simulink model "TosAeb"  (R2021a)
settings: Solver=FixedStepDiscrete  StartTime=0.0  StopTime=inf  FixedStep=0.01  RelTol=1e-3
undefined symbols (not defined in the file or in its folder): ACCModSts, AccSts1, AEB_ObjectMovingOrientation, AEB_ObjectMovingState, CN_BTCActive, CN_CurrentGearR, CN_CurrentGearR_DHT, CN_PTCActive, CN_VDCActive, CN_VSC_EspOff, CN_VSC_FCW_AEB_Error, CN_VSC_FCW_AEB_NoDispay, CN_VSC_FCW_AEB_PerfmDgdtn, CN_VSC_FCW_AEB_TempUnAvlbl, CN_VSC_VehStandstill, CNaccelAvoidMax, CNaccelAvoidMin, CNbrakePedalPress, CNcomfortStrLeftAccelMax, CNcomfortStrLeftAccelMin, CNcomfortStrRightAccelMax, CNcomfortStrRightAccelMin, CNDrvDoorOpen, CNDrvModeEco, CNDrvModeNorm, CNDrvModeOther, CNDrvModeSport, CNDrvSBRreminder, CNNull, CNobjInvalidID, CNPassDoorOpen, CNPassSBRreminder, CNPEBSAccelNegInf, CNPEBSAccelNull, CNPEBSTimeInf, CNPEBSTimeNull, CNSTOPMAX, CNSTOPNULL, CNsubjWidth, CNSwtOFF, CNSwtON, CNTTCMAX, CNTTCNULL, CNVehConfigAEBCycNone, CNVehConfigAEBNone, CNVehConfigAEBPedNone, CNVehConfigFCWNone, CNVehConfigJANone, CollisionSeverity, FALSE, FCWSnvty, JACycObjCalcInfoDefaultParams, JAPedObjCalcInfoDefaultParams, JAVehObjCalcInfoDefaultParams, latCycObjCalcInfoDefaultParams, latPedObjCalcInfoDefaultParams, longObjCalcInfoDefaultParams, ModSts, movingStatus, ObjectType ... (+1112)
libraries: simulink x3031, AEBSFunctionLib x50, Ta_Library x33, AEBSStateMachineLib x23, Mylibrary x12, simulink_extras x7, ObjSelect_lib x3, dspstat3 x2
(part 97 of 102)

== stateflow chart TosAeb/FcwAebJa_Step_sys/ALG_AEBSystem/ALG_AEBController/AEB_FuncCondCalcApp/PedFunctionCondCalc/pedPrefillSuppAborCond/pedPrefillSuppCondCalc/pedPrefillSituationRelActiveCondCalc/objLatVyCond4PrefillCalc/ObjectIsLaterallyMovingForPrefillCondCalc ==
  (default) -> (junction 4)
  (junction 4) -> (junction 5) [order 1] : [abs(vyCorr) < P_pedPrefillVyMaxThres]
  (junction 4) -> (junction 23) [order 2] : {
      | 	objLatVyCond4Prefill = false;
      | }
  (junction 5) -> (junction 7)
  (junction 7) -> (junction 9) [order 1] : [abs(dy)< P_pedPrefillDyInnerAreaThres]
  (junction 9) -> (junction 32)
  (junction 7) -> (junction 13) [order 2]
  (junction 32) -> (junction 34) [order 1] : [ObjectTypeCameraConfirmed == true]
  (junction 32) -> (junction 11) [order 2] : {
      | 	objLatVyCond4Prefill = ...
      | (abs(vyCorr) > P_pedPrefillVyInnerAreaThres4Radar);
      | }
  (junction 34) -> (junction 36) : {
      | 	objLatVyCond4Prefill = ...
      | (abs(vyCorr) > P_pedPrefillVyInnerAreaThres4Fusion);
      | }
  (junction 13) -> (junction 15) [order 1] : [abs(dy)<P_pedPrefillDyMiddleAreaThres]
  (junction 13) -> (junction 48) [order 2]
  (junction 15) -> (junction 39)
  (junction 39) -> (junction 41) [order 1] : [ObjectTypeCameraConfirmed == true]
  (junction 39) -> (junction 43) [order 2] : {
      | 	objLatVyCond4Prefill = ...
      | (abs(vyCorr) > P_pedPrefillVyMiddleAreaThres4Radar);
      | }
  (junction 41) -> (junction 17) : {
      | 	objLatVyCond4Prefill = ...
      | (abs(vyCorr) > P_pedPrefillVyMiddleAreaThres4Fusion);
      | }
  (junction 48) -> (junction 50) [order 1] : [ObjectTypeCameraConfirmed == true]
  (junction 48) -> (junction 19) [order 2] : {
      | 	objLatVyCond4Prefill = ...
      | (abs(vyCorr) > P_pedPrefillVyOuterAreaThres4Radar);
      | }
  (junction 50) -> (junction 46) : {
      | 	objLatVyCond4Prefill = ...
      | (abs(vyCorr) > P_pedPrefillVyOuterAreaThres4Fusion);
      | }
  (junction 36) -> (junction 11)
  (junction 11) -> (junction 17)
  (junction 19) -> (junction 23)
  (junction 46) -> (junction 19)
  (junction 43) -> (junction 46)
  (junction 17) -> (junction 43)
  (junction 23) -> (junction 26)
  data: ObjectTypeCameraConfirmed input; dy input; vyCorr input; P_pedPrefillVyMaxThres input; objLatVyCond4Prefill output; P_pedPrefillDyInnerAreaThres input; P_pedPrefillDyMiddleAreaThres input; P_pedPrefillVyInnerAreaThres4Fusion input; P_pedPrefillVyInnerAreaThres4Radar input; P_pedPrefillVyMiddleAreaThres4Fusion input; P_pedPrefillVyMiddleAreaThres4Radar input; P_pedPrefillVyOuterAreaThres4Fusion input; P_pedPrefillVyOuterAreaThres4Radar input

== stateflow chart TosAeb/FcwAebJa_Step_sys/ALG_AEBSystem/ALG_AEBController/AEB_FuncImpManagementApp/FuncBlockTimeCalc/tBlockTimeBrkCalc/BlockTimeCalc/BlockTimeCalc ==
  state A
    en,du:
    blockTime = 0;
  state B
    en:
    blockTime = PblockTime;
    du:
    blockTime -= Ts;
  (default) -> (junction 7)
  (junction 7) -> A [order 2]
  (junction 7) -> B [order 1] : [blockTimeTriggerCond == true]
  B -> A : [blockTime <= 0]
  A -> B : [blockTimeTriggerCond == true]
  data: blockTimeTriggerCond input; Ts input single; PblockTime input single; blockTime output single

== stateflow chart TosAeb/FcwAebJa_Step_sys/ALG_AEBSystem/ALG_AEBController/AEB_FuncImpManagementApp/FuncBlockTimeCalc/tBlockTimeBrkReadyCalc/BlockTimeCalc/BlockTimeCalc ==
  state A
    en,du:
    blockTime = 0;
  state B
    en:
    blockTime = PblockTime;
    du:
    blockTime -= Ts;
  (default) -> (junction 7)
  (junction 7) -> A [order 2]
  (junction 7) -> B [order 1] : [blockTimeTriggerCond == true]
  B -> A : [blockTime <= 0]
  A -> B : [blockTimeTriggerCond == true]
  data: blockTimeTriggerCond input; Ts input single; PblockTime input single; blockTime output single

== stateflow chart TosAeb/FcwAebJa_Step_sys/ALG_AEBSystem/ALG_AEBController/AEB_FuncImpManagementApp/FuncBlockTimeCalc/tBlockTimeWarningCalc/BlockTimeCalc/BlockTimeCalc ==
  state A
    en,du:
    blockTime = 0;
  state B
    en:
    blockTime = PblockTime;
    du:
    blockTime -= Ts;
  (default) -> (junction 7)
  (junction 7) -> A [order 2]
  (junction 7) -> B [order 1] : [blockTimeTriggerCond == true]
  B -> A : [blockTime <= 0]
  A -> B : [blockTimeTriggerCond == true]
  data: blockTimeTriggerCond input; Ts input single; PblockTime input single; blockTime output single

== stateflow chart TosAeb/FcwAebJa_Step_sys/ALG_AEBSystem/ALG_AEBController/AEB_FuncImpManagementApp/FuncManeuArbCalc/BrkReadyManeuArbCalc/BrkReadyTriggerJudge/ABALvlCalc/If Action Subsystem/ABALvlCalcFlow ==
  state ABATriggerState
    state ABALvl3
      en,du:
      ABALvl = uint8(3);
    state ABALvl2
      en,du:
      ABALvl = uint8(2);
    state ABALvl1
      en,du:
      ABALvl = uint8(1);
    (default) -> (junction 14)
    (junction 14) -> ABALvl3 [order 1] : [AEB_CollisionSeverity == CollisionSeverity.High]
    (junction 14) -> (junction 16) [order 2]
    (junction 58) -> ABALvl3
    (junction 56) -> (junction 58)
    ABALvl2 -> ABALvl3 : [AEB_CollisionSeverity == CollisionSeverity.High]
    (junction 16) -> ABALvl2 [order 1] : [AEB_CollisionSeverity == CollisionSeverity.Medium]
    (junction 16) -> (junction 19) [order 2]
    ABALvl1 -> ABALvl2 [order 2] : [AEB_CollisionSeverity == CollisionSeverity.Medium]
    (junction 19) -> ABALvl1
    ABALvl1 -> (junction 56) [order 1] : [AEB_CollisionSeverity == CollisionSeverity.High]
  state ABANoTriggerState
    en,du:
    ABALvl = 0;
  (default) -> ABANoTriggerState
  ABANoTriggerState -> ABATriggerState : [ABATrigger == true && ...
      | AEB_CollisionSeverity ~= CollisionSeverity.None]
  ABATriggerState -> ABANoTriggerState : [ABATrigger == false]
  data: ABATrigger input; AEB_CollisionSeverity input Enum: CollisionSeverity; ABALvl output uint8

== stateflow chart TosAeb/FcwAebJa_Step_sys/ALG_AEBSystem/ALG_AEBController/AEB_FuncImpManagementApp/FuncManeuArbCalc/WarningManeuArbCalc/WarningTriggerCalc/acuteWarningLvlCalcFlow ==
  state acuteWarningTriggerState
    state Lvl4
      en,du:
      actuteWarningLvl = uint8(4);
    state Lvl3
      en,du:
      actuteWarningLvl = uint8(3);
    state Lvl2
      en,du:
      actuteWarningLvl = uint8(2);
    state Lvl1
      en,du:
      actuteWarningLvl = uint8(1);
    (default) -> (junction 54)
    (junction 54) -> Lvl4 [order 1] : [subjVel > P_FIM_AcutewarningLvl4VehSpdMinThres ]
    (junction 54) -> (junction 56) [order 2]
    (junction 56) -> Lvl3 [order 1] : [subjVel > P_FIM_AcutewarningLvl3VehSpdMinThres]
    (junction 56) -> (junction 59) [order 2]
    (junction 59) -> Lvl2 [order 1] : [subjVel > P_FIM_AcutewarningLvl2VehSpdMinThres]
    (junction 59) -> (junction 62) [order 2]
    (junction 62) -> Lvl1
  state acuteWarningNoTrigger
    en,du:
    actuteWarningLvl = uint8(0);
  (default) -> acuteWarningNoTrigger
  acuteWarningNoTrigger -> acuteWarningTriggerState : [acuteWarningTrigger == true &&...
      | subjVel>=P_FIM_AcutewarningLvl1VehSpdMinThres&&...
      | subjVel<=P_FIM_AcutewarningLvl4VehSpdMaxThres]
  acuteWarningTriggerState -> acuteWarningNoTrigger : [acuteWarningTrigger == false]
  data: acuteWarningTrigger input boolean; subjVel input; actuteWarningLvl output uint8; P_FIM_AcutewarningLvl1VehSpdMinThres input; P_FIM_AcutewarningLvl2VehSpdMinThres input; P_FIM_AcutewarningLvl3VehSpdMinThres input; P_FIM_AcutewarningLvl4VehSpdMinThres input; P_FIM_AcutewarningLvl4VehSpdMaxThres input

== matlab function TosAeb/AEB_Objsel/TA_Processor/AEB_ObjSelect/TargetInfoCheck/Data_precess/TA_EffectCalculate/Obj_FilterFlgCalculate/JA_ObjFilter/CollisionProbCalc/Subsystem/Subsystem/SegmentComponents/LatPosition ==
  data: P0 input; y output single; P1 input; P2 input; P3 input; tau input; dy output single
function [y, dy] = fun(P0,P1,P2,P3,tau)
%#codegen

%TODO Description is missing 

%For performance reasons the original expression, below, has been rewritten  

%Original expressions 
% dx为x的对自变量tau的导数
% 式中x为距离，dx为速度
% 式中tau为x取值对应的概率值，p0，p1,p2,p3前系数值的和为"1"
%x=(1-tau)^3*P0 + 3*(1-tau)^2*tau*P1 + 3*(1-tau)*tau.^2*P2 + tau^3*P3;
%dx = -3*(1-tau)^2*P0 + 3*(3*tau^2 - 4*tau + 1)*P1 + 3*(2*tau - 3*tau^2)*P2 + 3*tau^2*P3; 

tauPov2 = tau*tau; 
tauPov3 = tau*tauPov2; 
x = (3*(P1 - P2) - P0 + P3)*tauPov3 + 3*(P0 - 2*P1 + P2)*tauPov2 + 3*(P1 - P0)*tau + P0; 
dx = (9*P1 - 3*P0 - 9*P2 + 3*P3)*tauPov2 + (6*P0 - 12*P1 + 6*P2)*tau - 3*P0 + 3*P1;
y = x;
dy = dx;

== matlab function TosAeb/AEB_Objsel/TA_Processor/AEB_ObjSelect/TargetInfoCheck/Data_precess/TA_EffectCalculate/Obj_FilterFlgCalculate/JA_ObjFilter/CollisionProbCalc/Subsystem/Subsystem/SegmentComponents/LongPosition ==
  data: P0 input; x output single; P1 input; P2 input; P3 input; tau input; dx output single
function [x,dx] = fcn(P0,P1,P2,P3,tau)
%#codegen

%TODO Description is missing 

%For performance reasons the original expression, below, has been rewritten  

%Original expressions 
% dx为x的对自变量tau的导数
% 式中x为距离，dx为速度
% 式中tau为x取值对应的概率值，p0，p1,p2,p3前系数值的和为"1"
%x=(1-tau)^3*P0 + 3*(1-tau)^2*tau*P1 + 3*(1-tau)*tau.^2*P2 + tau^3*P3;
%dx = -3*(1-tau)^2*P0 + 3*(3*tau^2 - 4*tau + 1)*P1 + 3*(2*tau - 3*tau^2)*P2 + 3*tau^2*P3; 

tauPov2 = tau*tau; 
tauPov3 = tau*tauPov2; 
x = (3*(P1 - P2) - P0 + P3)*tauPov3 + 3*(P0 - 2*P1 + P2)*tauPov2 + 3*(P1 - P0)*tau + P0; 
dx = (9*P1 - 3*P0 - 9*P2 + 3*P3)*tauPov2 + (6*P0 - 12*P1 + 6*P2)*tau - 3*P0 + 3*P1;

== stateflow chart TosAeb/AEB_Objsel/TA_Processor/AEB_ObjSelect/TargetInfoCheck/Data_precess/TA_EffectCalculate/Obj_FilterFlgCalculate/JA_ObjFilter/CollisionProbCalcFlow ==
  (default) -> (junction 11)
  (junction 11) -> (junction 2) : {tPredictTime = 0;}
  (junction 2) -> (junction 6) [order 1] : [tPredictTime <= P_JaPedCA_PreCollisionPredTimeMax]
  (junction 6) -> (junction 8) : {
      | 	tPredictTimeOut = tPredictTime;
      | 	CollisionProbFunc;
      | }
  (junction 8) -> (junction 2) [order 2] : {
      | 	tPredictTime += P_JaPedCA_PreCollisionStepTime;
      | }
  (junction 2) -> (junction 13) [order 2] : {
      |   TTC = single(256);
      | }
  (junction 8) -> (junction 22) [order 1] : [preCollisionProb == true]
  (junction 22) -> (junction 13) : {
      |   TTC = tPredictTimeOut;
      | }
  (junction 13) -> (junction 25)
  data: TTC output single; P_JaPedCA_PreCollisionStepTime input single; P_JaPedCA_PreCollisionPredTimeMax input single; tPredictTimeOut output single; tPredictTime local single; preCollisionProb input
  events: CollisionProbFunc output FUNCTION_CALL_EVENT

== stateflow chart TosAeb/FcwAebJa_Step_sys/ALG_AEBSystem/ALG_AEBController/AEB_FuncImpManagementApp/FuncManeuArbCalc/brkFuncManeuArbCalc/axTgtReqValueManeuCalc/AEBTriggerManeuCalc ==
  state AEBQuitState
    en:
    timeCounter = 0;
    AEBTriggerManeu = true;
    %axTgtReqValueIntial = axTgtReqValue;
    du:
    AEBTriggerManeu = true;
    timeCounter += Ts;
    axTgtReqValueManeu = axTgtReqValueOptCalc(Ts,axJerkQuit,axTgtReqValueManeuK1);
  state AEBNormalState
    en,du:
    timeCounter = 0;
    AEBTriggerManeu= AEBTrigger;
    axTgtReqValueManeu = axTgtReqValue;
    %exit:
    %axTgtReqValueIntial = axTgtReqValueK1;
  state axTgtReqValueManeu = axTgtReqValueOptCalc(Ts,axJerkQuit,axTgtReqValueManeuK1) [function]
  (default) -> (junction 7)
  (junction 7) -> AEBNormalState [order 2]
  (junction 7) -> AEBQuitState [order 1] : [AEBQuitFlg == true]
  AEBQuitState -> AEBNormalState : [timeCounter >= PmaxQuitTime || ...
      | abs(axTgtReqValueManeu) < 1e-6]
  AEBNormalState -> AEBQuitState : [AEBQuitFlg == true]
  data: Ts function_input single; axJerkQuit function_input single; axTgtReqValueManeuK1 function_input single; axTgtReqValueManeu function_output; AEBQuitFlg input; AEBTrigger input; axTgtReqValue input single; Ts input single; timeCounter local single; PmaxQuitTime input single; AEBTriggerManeu output boolean; axTgtReqValueManeu output single; axJerkQuit input single; axTgtReqValueManeuK1 input

== stateflow chart TosAeb/FcwAebJa_Step_sys/ALG_AEBSystem/ALG_AEBController/AEB_FuncImpManagementApp/FuncManeuArbCalc/brkFuncManeuArbCalc/brkManeuAborCalc/ESPRelAborCondCalc/ESPRelAborCondFlow ==
  (default) -> (junction 6)
  (junction 6) -> (junction 7) [order 1] : [AEBIBK1 == true]
  (junction 6) -> (junction 18) [order 2]
  (junction 7) -> (junction 13) : {
      | 	ESPRelAborCond = ((AEBIB == false) &&(AEBBA == false));
      | }
  (junction 18) -> (junction 20) [order 1] : [AEBBAK1 == true]
  (junction 18) -> (junction 24) [order 2] : {
      | 	ESPRelAborCond = false;
      | }
  (junction 20) -> (junction 22) : {
      | 	ESPRelAborCond = (AEBBA == false);
      | }
  (junction 22) -> (junction 24)
  (junction 13) -> (junction 22)
  (junction 24) -> (junction 28)
  data: AEBBA input; AEBBAK1 input; AEBIB input; AEBIBK1 input; ESPRelAborCond output

== stateflow chart TosAeb/FcwAebJa_Step_sys/ALG_AEBSystem/ALG_AEBController/AEB_FuncImpManagementApp/FuncManeuArbCalc/brkFuncManeuArbCalc/brkManeuAborCalc/VelReduceMaxCond/vxTriggerCalc ==
  state VelReduceMaxCondCalc [function]
    (default) -> (junction 38)
    (junction 38) -> (junction 40) [order 1] : [EBAFuncSts == ModSts.ActiveState]
    (junction 38) -> (junction 63) [order 2]
    (junction 40) -> (junction 42)
    (junction 63) -> (junction 65) [order 1] : [vxTrigger < P_FIM_AEBVelDecMaxThres]
    (junction 42) -> (junction 45) [order 1] : [vxTrigger < P_FIM_EBAVelDecMaxThres]
    (junction 63) -> (junction 69) [order 2] : {
        | subjVelReduced = ...
        | vxTrigger - P_FIM_AEBVelDecMaxThres;
        | }
    (junction 65) -> (junction 67) : {
        |   subjVelReduced  = 0;
        | }
    (junction 42) -> (junction 49) [order 2] : {
        | subjVelReduced = ...
        | vxTrigger - P_FIM_EBAVelDecMaxThres;
        | }
    (junction 45) -> (junction 47) : {
        |   subjVelReduced  = 0;
        | }
    (junction 67) -> (junction 69)
    (junction 69) -> (junction 88)
    (junction 47) -> (junction 49)
    (junction 49) -> (junction 86)
    (junction 86) -> (junction 88)
    (junction 88) -> (junction 52)
    (junction 52) -> (junction 54) [order 1] : [subjVel < subjVelReduced]
    (junction 52) -> (junction 58) [order 2] : {
        |   VelReduceMaxCond = false;
        | }
    (junction 54) -> (junction 56) : {
        |   VelReduceMaxCond = true;
        | }
    (junction 56) -> (junction 58)
    (junction 58) -> (junction 61)
  state PassiveState
    en,du:
    %vxTrigger = 0;
    VelReduceMaxCond = false;
  state TriggerState
    en:
    vxTrigger = max(subjVel,0);
    du:
    VelReduceMaxCondCalc();
  (default) -> PassiveState
  PassiveState -> TriggerState : [AEBTrigger == true]
  TriggerState -> PassiveState : [AEBTrigger == false]
  data: AEBTrigger input boolean; subjVel input single; EBAFuncSts input Enum: ModSts; vxTrigger local single; VelReduceMaxCond output boolean; P_FIM_EBAVelDecMaxThres input single; P_FIM_AEBVelDecMaxThres input single; subjVelReduced local single

== stateflow chart TosAeb/FcwAebJa_Step_sys/ALG_AEBSystem/ALG_AEBController/AEB_LongTgtInfoCalc/LongObjCalcInfoCalc/AEBS_LongCollisionAssesApp/DriverReactionTimeCalc/ACCStateMonitor/ACCStateMonitor ==
  state NormalState
    en,du:
    NormalStateFunc();
  state NormalStateFunc [function]
    (default) -> (junction 86)
    (junction 86) -> (junction 84) [order 1] : [ACCstateK1 == 0 &&...
        | ACCstate == 1]
    (junction 86) -> (junction 80) [order 2] : {
        | 	ACCMonitor = false;
        | }
    (junction 84) -> (junction 79) : {
        | ACCMonitor = true;
        | }
    (junction 79) -> (junction 80)
    (junction 80) -> (junction 89)
  state CounterState
    en:
    timesincelastACC = 0;
    du:
    timesincelastACC +=  Ts;
    CounterStateFunc();
  state CounterStateFunc [function]
    (default) -> (junction 70)
    (junction 70) -> (junction 62) [order 1] : [timesincelastACC > CNreactionTimetimesincelastACCMAX]
    (junction 70) -> (junction 69) [order 2] : %do nothing
    (junction 62) -> (junction 61) : {
        | timesincelastACC = CNreactionTimetimesincelastACCMAX;
        | }
    (junction 61) -> (junction 69)
    (junction 69) -> (junction 68)
    (junction 68) -> (junction 60) [order 1] : [timesincelastACC < PreactionTimeProlongationTimeACC]
    (junction 68) -> (junction 66) [order 2] : {
        | ACCMonitor = false;
        | }
    (junction 60) -> (junction 67) : {
        | ACCMonitor = true;
        | }
    (junction 67) -> (junction 66)
    (junction 66) -> (junction 56)
  (default) -> NormalState
  NormalState -> CounterState : [ACCstateK1 == true &&...
      | ACCstate == false]
  CounterState -> NormalState : [timesincelastACC >= PreactionTimeProlongationTimeACC]
  data: ACCstate input; ACCstateK1 input; PreactionTimeProlongationTimeACC input; timesincelastACC output double; ACCMonitor output boolean; Ts input; CNreactionTimetimesincelastACCMAX parameter double

== stateflow chart TosAeb/FcwAebJa_Step_sys/ALG_AEBSystem/ALG_AEBController/AEB_LongTgtInfoCalc/LongObjCalcInfoCalc/AEBS_LongCollisionAssesApp/DriverReactionTimeCalc/ReationTimeCalc1/ReactionTimeLevel1Calc ==
  note: Diver Reaction Time Calculation
  note: Note:HMISetting Code
      | CNHMIEarly: 0
      | CNHMIMiddle: 1
      | CNHMILate: 2
  (default) -> (junction 7)
  (junction 7) -> (junction 8) [order 1] : [FcwHMISetting == FCWSnvty.HMISettingEarly]
  (junction 7) -> (junction 19) [order 2]
  (junction 8) -> (junction 10)
  (junction 19) -> (junction 21) [order 1] : [FcwHMISetting == FCWSnvty.HMISettingMiddle]
  (junction 19) -> (junction 39) [order 2] : %HMISetting == CNHMILate
  (junction 21) -> (junction 23)
  (junction 39) -> (junction 41) [order 1] : [driverDetectAsActiveFlag == true]
  (junction 23) -> (junction 32) [order 1] : [driverDetectAsActiveFlag == true]
  (junction 10) -> (junction 12) [order 1] : [driverDetectAsActiveFlag == true]
  (junction 39) -> (junction 45) [order 2] : {
      | 	tempDiverReactionTimeLvl1 = ...
      | 	PreactionTimeLvl1HMILate;
      | }
  (junction 41) -> (junction 43) : {
      | 	tempDiverReactionTimeLvl1 = ...
      | 	PreactionTimeAttentiveDriverLvl1HMILate;
      | }
  (junction 23) -> (junction 30) [order 2] : {
      | 	tempDiverReactionTimeLvl1 = ...
      | 	PreactionTimeLvl1HMIMiddle;
      | }
  (junction 32) -> (junction 34) : {
      | 	tempDiverReactionTimeLvl1 = ...
      | 	PreactionTimeAttentiveDriverLvl1HMIMiddle;
      | }
  (junction 10) -> (junction 16) [order 2] : {
      | 	tempDiverReactionTimeLvl1 = ...
      | 	PreactionTimeLvl1HMIEarly;
      | }
  (junction 12) -> (junction 14) : {
      | 	tempDiverReactionTimeLvl1 = ...
      | 	PreactionTimeAttentiveDriverLvl1HMIEarly;
      | }
  (junction 14) -> (junction 16)
  (junction 34) -> (junction 30)
  (junction 16) -> (junction 56)
  (junction 30) -> (junction 52)
  (junction 43) -> (junction 45)
  (junction 45) -> (junction 50)
  (junction 56) -> (junction 52)
  (junction 52) -> (junction 50)
  (junction 50) -> (junction 48) : {
      | 	diverReactionTimeLvl1 = tempDiverReactionTimeLvl1;
      |  }
  data: FcwHMISetting input; driverDetectAsActiveFlag input; PreactionTimeLvl1HMIEarly input; PreactionTimeLvl1HMIMiddle input; PreactionTimeLvl1HMILate input; PreactionTimeAttentiveDriverLvl1HMIEarly input; PreactionTimeAttentiveDriverLvl1HMIMiddle input; PreactionTimeAttentiveDriverLvl1HMILate input; diverReactionTimeLvl1 output; tempDiverReactionTimeLvl1 local double

== stateflow chart TosAeb/FcwAebJa_Step_sys/ALG_AEBSystem/ALG_AEBController/AEB_LongTgtInfoCalc/LongObjCalcInfoCalc/AEBS_LongCollisionAssesApp/LatCollisionAvoidCalc/AccelLatAvoidCalc4SO/accelLatAvoidLeftCalc/CalculateAccelSteeringLeft/CalculateAccelSteeringLeftFlow ==
  (default) -> (junction 2)
  (junction 2) -> (junction 3) [order 1] : [tempFlg]
  (junction 2) -> (junction 23) [order 2] : {
      | 	accelSteeringLeft  = CNcomfortStrLeftAccelMax;
      | }
  (junction 3) -> (junction 21) : {
      | 	tempData = PcomfortStrLatDistMinSO + dyRelTargetObjCalc;
      | }
  (junction 21) -> (junction 29) [order 1] : [tempData <= 0]
  (junction 21) -> (junction 33) [order 2] : {
      | 	accelSteeringLeftCalcFunc;
      | 	accelSteeringLeft = accelSteeringLeftIn;
      | }
  (junction 29) -> (junction 31) : {
      | 	accelSteeringLeft  = CNcomfortStrLeftAccelMin;
      | }
  (junction 33) -> (junction 23)
  (junction 31) -> (junction 33)
  (junction 23) -> (junction 46)
  data: tempFlg input; PcomfortStrLatDistMinSO input; dyRelTargetObjCalc input; accelSteeringLeftIn input; accelSteeringLeft output; tempData local; CNcomfortStrLeftAccelMax parameter; CNcomfortStrLeftAccelMin parameter
  events: accelSteeringLeftCalcFunc output FUNCTION_CALL_EVENT

== stateflow chart TosAeb/FcwAebJa_Step_sys/ALG_AEBSystem/ALG_AEBController/AEB_LongTgtInfoCalc/LongObjCalcInfoCalc/AEBS_LongCollisionAssesApp/LatCollisionAvoidCalc/AccelLatAvoidCalc4SO/accelLatAvoidRightCalc/CalculateAccelSteeringRight/CalculateAccelSteeringLeftFlow ==
  (default) -> (junction 2)
  (junction 2) -> (junction 3) [order 1] : [tempFlg]
  (junction 2) -> (junction 23) [order 2] : {
      | 	accelSteeringRight  = CNcomfortStrRightAccelMin;
      | }
  (junction 3) -> (junction 21) : {
      | 	tempData = PcomfortStrLatDistMinSO - dyRelTargetObjCalc;
      | }
  (junction 21) -> (junction 29) [order 1] : [tempData <= 0]
  (junction 21) -> (junction 33) [order 2] : {
      | 	accelSteeringRightCalcFunc;
      | 	accelSteeringRight = accelSteeringRightIn;
      | }
  (junction 29) -> (junction 31) : {
      | 	accelSteeringRight  = CNcomfortStrRightAccelMax;
      | }
  (junction 33) -> (junction 23)
  (junction 31) -> (junction 33)
  (junction 23) -> (junction 46)
  data: tempFlg input; PcomfortStrLatDistMinSO input; dyRelTargetObjCalc input; tempData local; CNcomfortStrRightAccelMax parameter; CNcomfortStrRightAccelMin parameter; accelSteeringRightIn input; accelSteeringRight output
  events: accelSteeringRightCalcFunc output FUNCTION_CALL_EVENT

== stateflow chart TosAeb/FcwAebJa_Step_sys/ALG_AEBSystem/ALG_AEBController/AEB_LongTgtInfoCalc/LongObjCalcInfoCalc/AEBS_LongCollisionAssesApp/LongCollisionAvoidAssess/HighLvlBrkCalc/HighLvlBrkCalc4Brake/accelAvoidHighLvlBrkSituation1/accelAvoidLowLvlBrkCalc/AccelAvoidSituationJudge ==
  (default) -> (junction 16)
  (junction 16) -> (junction 17) [order 1] : [vx < 0]
  (junction 16) -> (junction 27) [order 2]
  (junction 17) -> (junction 74)
  (junction 74) -> (junction 76) [order 1] : [axAbsObj  < 0]
  (junction 27) -> (junction 29) [order 1] : [ axAbsObj  < 0]
  (junction 74) -> (junction 25) [order 2] : {
      | 	send(Situation2);
      | }
  (junction 76) -> (junction 19) : {
      | 	send(Situation1);
      | }
  (junction 27) -> (junction 33) [order 2] : {
      | 	send(Situation4);
      | }
  (junction 29) -> (junction 31) : {
      | 	send(Situation3);
      | }
  (junction 31) -> (junction 33)
  (junction 25) -> (junction 31)
  (junction 19) -> (junction 25)
  (junction 33) -> (junction 86)
  data: vx input; axAbsObj input
  events: Situation1 output FUNCTION_CALL_EVENT; Situation2 output FUNCTION_CALL_EVENT; Situation3 output FUNCTION_CALL_EVENT; Situation4 output FUNCTION_CALL_EVENT

== stateflow chart TosAeb/FcwAebJa_Step_sys/ALG_AEBSystem/ALG_AEBController/AEB_LongTgtInfoCalc/LongObjCalcInfoCalc/AEBS_LongCollisionAssesApp/LongCollisionAvoidAssess/HighLvlBrkCalc/HighLvlBrkCalc4Brake/accelAvoidHighLvlBrkSituation1/accelAvoidLowLvlBrkCalc2/AccelAvoidSituationJudge ==
  (default) -> (junction 16)
  (junction 16) -> (junction 17) [order 1] : [vxRelTargetObjCalc < 0 &&...
      |  axAbsTargetObjCalc  < 0]
  (junction 16) -> (junction 21) [order 2]
  (junction 17) -> (junction 19) : {send(Situation1);}
  (junction 21) -> (junction 23) [order 1] : [vxRelTargetObjCalc < 0 &&...
      |  axAbsTargetObjCalc  >= 0]
  (junction 21) -> (junction 27) [order 2]
  (junction 23) -> (junction 25) : {send(Situation2);}
  (junction 27) -> (junction 29) [order 1] : [vxRelTargetObjCalc > 0 &&...
      |  axAbsTargetObjCalc  < 0]
  (junction 27) -> (junction 33) [order 2] : {send(Situation4);}
  (junction 29) -> (junction 31) : {send(Situation3);}
  (junction 31) -> (junction 33)
  (junction 25) -> (junction 31)
  (junction 19) -> (junction 25)
  (junction 33) -> (junction 39)
  data: vxRelTargetObjCalc input; axAbsTargetObjCalc input
  events: Situation1 output FUNCTION_CALL_EVENT; Situation2 output FUNCTION_CALL_EVENT; Situation3 output FUNCTION_CALL_EVENT; Situation4 output FUNCTION_CALL_EVENT

== stateflow chart TosAeb/FcwAebJa_Step_sys/ALG_AEBSystem/ALG_AEBInput/LongObjInfoMap/ObjType_Subsystem/ObjectType ==
  (default) -> (junction 17)
  (junction 17) -> (junction 18) [order 1] : [ObjCar==1]
  (junction 17) -> (junction 19) [order 2]
  (junction 18) -> (junction 23) : {ObjType = 2;}
  (junction 19) -> (junction 20) [order 1] : [ObjTruck ==1]
  (junction 19) -> (junction 26) [order 2]
  (junction 20) -> (junction 22) : {
      | 	ObjType=3;
      | }
  (junction 26) -> (junction 27) [order 1] : [ObjPed==1]
  (junction 26) -> (junction 83) [order 2]
  (junction 27) -> (junction 29) : {
      | 	ObjType=6;
      | }
  (junction 83) -> (junction 85) [order 1] : [ObjMotor==1]
  (junction 83) -> (junction 91) [order 2]
  (junction 85) -> (junction 87) : {ObjType=4;}
  (junction 91) -> (junction 93) [order 1] : [ObjBicycle==1]
  (junction 91) -> (junction 100) [order 2]
  (junction 93) -> (junction 95) : {ObjType=5;}
  (junction 100) -> (junction 102) [order 1] : [ObjGeneral==1]
  (junction 100) -> (junction 21) [order 2] : {ObjType=1;}
  (junction 102) -> (junction 104) : {ObjType=7;}
  (junction 104) -> (junction 21)
  (junction 95) -> (junction 104)
  (junction 87) -> (junction 95)
  (junction 29) -> (junction 87)
  (junction 22) -> (junction 29)
  (junction 23) -> (junction 22)
  (junction 21) -> (junction 25)
  data: ObjCar input; ObjTruck input; ObjPed input; ObjType output uint8; ObjMotor input; ObjBicycle input; ObjGeneral input; Unknown input

== stateflow chart TosAeb/FcwAebJa_Step_sys/ALG_AEBSystem/ALG_AEBController/AEB_LongTgtInfoCalc/LongObjCalcInfoCalc/AEBS_LongCollisionAssesApp/LongCollisionAvoidAssess/HighLvlBrkCalc/HighLvlBrkCalc4Brake/accelAvoidHighLvlBrkSituation2/accelAvoidHighLvlBrkCalc/If Action Subsystem1/accelAvoidLowLvlBrkCalc/AccelAvoidSituationJudge ==
  (default) -> (junction 16)
  (junction 16) -> (junction 17) [order 1] : [vx < 0]
  (junction 16) -> (junction 27) [order 2]
  (junction 17) -> (junction 74)
  (junction 74) -> (junction 76) [order 1] : [axAbsObj  < 0]
  (junction 27) -> (junction 29) [order 1] : [ axAbsObj  < 0]
  (junction 74) -> (junction 25) [order 2] : {
      | 	send(Situation2);
      | }
  (junction 76) -> (junction 19) : {
      | 	send(Situation1);
      | }
  (junction 27) -> (junction 33) [order 2] : {
      | 	send(Situation4);
      | }
  (junction 29) -> (junction 31) : {
      | 	send(Situation3);
      | }
  (junction 31) -> (junction 33)
  (junction 25) -> (junction 31)
  (junction 19) -> (junction 25)
  (junction 33) -> (junction 86)
  data: vx input; axAbsObj input
  events: Situation1 output FUNCTION_CALL_EVENT; Situation2 output FUNCTION_CALL_EVENT; Situation3 output FUNCTION_CALL_EVENT; Situation4 output FUNCTION_CALL_EVENT

== stateflow chart TosAeb/FcwAebJa_Step_sys/ALG_AEBSystem/ALG_AEBController/AEB_LongTgtInfoCalc/LongObjCalcInfoCalc/AEBS_LongCollisionAssesApp/LongCollisionAvoidAssess/HighLvlBrkCalc/HighLvlBrkCalc4Brake/accelAvoidHighLvlBrkSituation2/accelAvoidHighLvlBrkCalc/If Action Subsystem1/accelAvoidLowLvlBrkCalc1/AccelAvoidSituationJudge ==
  (default) -> (junction 16)
  (junction 16) -> (junction 17) [order 1] : [vxRelTargetObjCalc < 0 &&...
      |  axAbsTargetObjCalc  < 0]
  (junction 16) -> (junction 21) [order 2]
  (junction 17) -> (junction 19) : {send(Situation1);}
  (junction 21) -> (junction 23) [order 1] : [vxRelTargetObjCalc < 0 &&...
      |  axAbsTargetObjCalc  >= 0]
  (junction 21) -> (junction 27) [order 2]
  (junction 23) -> (junction 25) : {send(Situation2);}
  (junction 27) -> (junction 29) [order 1] : [vxRelTargetObjCalc > 0 &&...
      |  axAbsTargetObjCalc  < 0]
  (junction 27) -> (junction 33) [order 2] : {send(Situation4);}
  (junction 29) -> (junction 31) : {send(Situation3);}
  (junction 31) -> (junction 33)
  (junction 25) -> (junction 31)
  (junction 19) -> (junction 25)
  (junction 33) -> (junction 39)
  data: vxRelTargetObjCalc input; axAbsTargetObjCalc input
  events: Situation1 output FUNCTION_CALL_EVENT; Situation2 output FUNCTION_CALL_EVENT; Situation3 output FUNCTION_CALL_EVENT; Situation4 output FUNCTION_CALL_EVENT

== stateflow chart TosAeb/FcwAebJa_Step_sys/ALG_AEBSystem/ALG_AEBController/AEB_LongTgtInfoCalc/LongObjCalcInfoCalc/AEBS_LongCollisionAssesApp/LongCollisionAvoidAssess/HighLvlBrkCalc/HighLvlBrkCalc4Brake/accelAvoidHighLvlBrkSituation2/accelAvoidHighLvlBrkCalc/tObjStopCalc/StopTimeFlow ==
  (default) -> (junction 2)
  (junction 2) -> (junction 3) [order 1] : [accelRel == 0]
  (junction 2) -> (junction 14) [order 2]
  (junction 3) -> (junction 5)
  (junction 14) -> (junction 16) [order 1] : [(accelRel > 0 && velRel < 0)||...
      | (accelRel < 0 && velRel >= 0)]
  (junction 5) -> (junction 7) [order 1] : [velRel == 0]
  (junction 14) -> (junction 20) [order 2] : {
      | 	tStop = CNSTOPNULL;
      |  }
  (junction 16) -> (junction 18) : {
      | 	tStopFuncCalc;
      | 	tStop = tStopIn;
      | }
  (junction 5) -> (junction 11) [order 2] : {
      | 	tStop = CNSTOPMAX;
      | }
  (junction 7) -> (junction 9) : {
      | 	tStop = CNSTOPNULL;
      | }
  (junction 18) -> (junction 20)
  (junction 11) -> (junction 18)
  (junction 9) -> (junction 11)
  (junction 20) -> (junction 24)
  data: tStop output single; CNSTOPNULL constant single; CNSTOPMAX constant single; accelRel input; velRel input; tStopIn input
  events: tStopFuncCalc output FUNCTION_CALL_EVENT

== stateflow chart TosAeb/FcwAebJa_Step_sys/ALG_AEBSystem/ALG_AEBController/AEB_LongTgtInfoCalc/LongObjCalcInfoCalc/AEBS_LongCollisionAssesApp/LongCollisionAvoidAssess/HighLvlBrkCalc/HighLvlBrkCalc4Brake/accelAvoidHighLvlBrkSituation3/If Action Subsystem1/accelAvoidHighLvlBrkCalc/accelAvoidLowLvlBrkCalc/AccelAvoidSituationJudge ==
  (default) -> (junction 16)
  (junction 16) -> (junction 17) [order 1] : [vx < 0]
  (junction 16) -> (junction 27) [order 2]
  (junction 17) -> (junction 74)
  (junction 74) -> (junction 76) [order 1] : [axAbsObj  < 0]
  (junction 27) -> (junction 29) [order 1] : [ axAbsObj  < 0]
  (junction 74) -> (junction 25) [order 2] : {
      | 	send(Situation2);
      | }
  (junction 76) -> (junction 19) : {
      | 	send(Situation1);
      | }
  (junction 27) -> (junction 33) [order 2] : {
      | 	send(Situation4);
      | }
  (junction 29) -> (junction 31) : {
      | 	send(Situation3);
      | }
  (junction 31) -> (junction 33)
  (junction 25) -> (junction 31)
  (junction 19) -> (junction 25)
  (junction 33) -> (junction 86)
  data: vx input; axAbsObj input
  events: Situation1 output FUNCTION_CALL_EVENT; Situation2 output FUNCTION_CALL_EVENT; Situation3 output FUNCTION_CALL_EVENT; Situation4 output FUNCTION_CALL_EVENT

== stateflow chart TosAeb/FcwAebJa_Step_sys/ALG_AEBSystem/ALG_AEBController/AEB_LongTgtInfoCalc/LongObjCalcInfoCalc/AEBS_LongCollisionAssesApp/LongCollisionAvoidAssess/HighLvlBrkCalc/HighLvlBrkCalc4Brake/accelAvoidHighLvlBrkSituation3/If Action Subsystem1/accelAvoidHighLvlBrkCalc/accelAvoidLowLvlBrkCalc1/AccelAvoidSituationJudge ==
  (default) -> (junction 16)
  (junction 16) -> (junction 17) [order 1] : [vxRelTargetObjCalc < 0 &&...
      |  axAbsTargetObjCalc  < 0]
  (junction 16) -> (junction 21) [order 2]
  (junction 17) -> (junction 19) : {send(Situation1);}
  (junction 21) -> (junction 23) [order 1] : [vxRelTargetObjCalc < 0 &&...
      |  axAbsTargetObjCalc  >= 0]
  (junction 21) -> (junction 27) [order 2]
  (junction 23) -> (junction 25) : {send(Situation2);}
  (junction 27) -> (junction 29) [order 1] : [vxRelTargetObjCalc > 0 &&...
      |  axAbsTargetObjCalc  < 0]
  (junction 27) -> (junction 33) [order 2] : {send(Situation4);}
  (junction 29) -> (junction 31) : {send(Situation3);}
  (junction 31) -> (junction 33)
  (junction 25) -> (junction 31)
  (junction 19) -> (junction 25)
  (junction 33) -> (junction 39)
  data: vxRelTargetObjCalc input; axAbsTargetObjCalc input
  events: Situation1 output FUNCTION_CALL_EVENT; Situation2 output FUNCTION_CALL_EVENT; Situation3 output FUNCTION_CALL_EVENT; Situation4 output FUNCTION_CALL_EVENT

== stateflow chart TosAeb/FcwAebJa_Step_sys/ALG_AEBSystem/ALG_AEBController/AEB_LongTgtInfoCalc/LongObjCalcInfoCalc/AEBS_LongCollisionAssesApp/LongCollisionAvoidAssess/HighLvlBrkCalc/HighLvlBrkCalc4Brake/accelAvoidHighLvlBrkSituationJudge ==
  note: NOTE:
      | Stuation1:	tReaction == 0
      | Situation2:	tReaction < min(tEgoStop,tObjStop)
      | Situation3:	tObjStop<tReaction<=tEgoStop
      | Stuation4:	tEgoStop<tReaction<=tObjStop
      | Situation5: tReaction > max(tEgoStop,tObjStop)
  (default) -> (junction 2)
  (junction 2) -> (junction 3) [order 1] : [tReaction == 0]
  (junction 2) -> (junction 7) [order 2]
  (junction 3) -> (junction 5) : {send(Situation1);}
  (junction 7) -> (junction 9) [order 1] : [tReaction<=tEgoStop &&...
      | tReaction<=tObjStop]
  (junction 7) -> (junction 13) [order 2]
  (junction 9) -> (junction 11) : {send(Situation2);}
  (junction 13) -> (junction 15) [order 1] : [tReaction<=tEgoStop && ...
      | tReaction>tObjStop]
  (junction 13) -> (junction 19) [order 2]
  (junction 15) -> (junction 17) : {send(Situation3);}
  (junction 19) -> (junction 21) [order 1] : [tReaction>tEgoStop && ...
      | tReaction<=tObjStop ]
  (junction 19) -> (junction 25) [order 2] : {send(Situation5);}
  (junction 21) -> (junction 23) : {send(Situation4);}
  (junction 23) -> (junction 25)
  (junction 17) -> (junction 23)
  (junction 11) -> (junction 17)
  (junction 5) -> (junction 11)
  (junction 25) -> (junction 28)
  data: tEgoStop input; tObjStop input; tReaction input
  events: Situation1 output FUNCTION_CALL_EVENT; Situation2 output FUNCTION_CALL_EVENT; Situation3 output FUNCTION_CALL_EVENT; Situation4 output FUNCTION_CALL_EVENT; Situation5 output FUNCTION_CALL_EVENT

== stateflow chart TosAeb/FcwAebJa_Step_sys/ALG_AEBSystem/ALG_AEBController/AEB_LongTgtInfoCalc/LongObjCalcInfoCalc/AEBS_LongCollisionAssesApp/LongCollisionAvoidAssess/HighLvlBrkCalc/HighLvlBrkCalc4Warning/accelAvoidHighLvlBrkSituation1/accelAvoidLowLvlBrkCalc/AccelAvoidSituationJudge ==
  (default) -> (junction 16)
  (junction 16) -> (junction 17) [order 1] : [vx < 0]
  (junction 16) -> (junction 27) [order 2]
  (junction 17) -> (junction 74)
  (junction 74) -> (junction 76) [order 1] : [axAbsObj  < 0]
  (junction 27) -> (junction 29) [order 1] : [ axAbsObj  < 0]
  (junction 74) -> (junction 25) [order 2] : {
      | 	send(Situation2);
      | }
  (junction 76) -> (junction 19) : {
      | 	send(Situation1);
      | }
  (junction 27) -> (junction 33) [order 2] : {
      | 	send(Situation4);
      | }
  (junction 29) -> (junction 31) : {
      | 	send(Situation3);
      | }
  (junction 31) -> (junction 33)
  (junction 25) -> (junction 31)
  (junction 19) -> (junction 25)
  (junction 33) -> (junction 86)
  data: vx input; axAbsObj input
  events: Situation1 output FUNCTION_CALL_EVENT; Situation2 output FUNCTION_CALL_EVENT; Situation3 output FUNCTION_CALL_EVENT; Situation4 output FUNCTION_CALL_EVENT

== stateflow chart TosAeb/FcwAebJa_Step_sys/ALG_AEBSystem/ALG_AEBController/AEB_LongTgtInfoCalc/LongObjCalcInfoCalc/AEBS_LongCollisionAssesApp/LongCollisionAvoidAssess/HighLvlBrkCalc/HighLvlBrkCalc4Warning/accelAvoidHighLvlBrkSituation1/accelAvoidLowLvlBrkCalc2/AccelAvoidSituationJudge ==
  (default) -> (junction 16)
  (junction 16) -> (junction 17) [order 1] : [vxRelTargetObjCalc < 0 &&...
      |  axAbsTargetObjCalc  < 0]
  (junction 16) -> (junction 21) [order 2]
  (junction 17) -> (junction 19) : {send(Situation1);}
  (junction 21) -> (junction 23) [order 1] : [vxRelTargetObjCalc < 0 &&...
      |  axAbsTargetObjCalc  >= 0]
  (junction 21) -> (junction 27) [order 2]
  (junction 23) -> (junction 25) : {send(Situation2);}
  (junction 27) -> (junction 29) [order 1] : [vxRelTargetObjCalc > 0 &&...
      |  axAbsTargetObjCalc  < 0]
  (junction 27) -> (junction 33) [order 2] : {send(Situation4);}
  (junction 29) -> (junction 31) : {send(Situation3);}
  (junction 31) -> (junction 33)
  (junction 25) -> (junction 31)
  (junction 19) -> (junction 25)
  (junction 33) -> (junction 39)
  data: vxRelTargetObjCalc input; axAbsTargetObjCalc input
  events: Situation1 output FUNCTION_CALL_EVENT; Situation2 output FUNCTION_CALL_EVENT; Situation3 output FUNCTION_CALL_EVENT; Situation4 output FUNCTION_CALL_EVENT

== stateflow chart TosAeb/FcwAebJa_Step_sys/ALG_AEBSystem/ALG_AEBController/AEB_LongTgtInfoCalc/LongObjCalcInfoCalc/AEBS_LongCollisionAssesApp/LongCollisionAvoidAssess/HighLvlBrkCalc/HighLvlBrkCalc4Warning/accelAvoidHighLvlBrkSituation2/accelAvoidHighLvlBrkCalc/If Action Subsystem1/accelAvoidLowLvlBrkCalc/AccelAvoidSituationJudge ==
  (default) -> (junction 16)
  (junction 16) -> (junction 17) [order 1] : [vx < 0]
  (junction 16) -> (junction 27) [order 2]
  (junction 17) -> (junction 74)
  (junction 74) -> (junction 76) [order 1] : [axAbsObj  < 0]
  (junction 27) -> (junction 29) [order 1] : [ axAbsObj  < 0]
  (junction 74) -> (junction 25) [order 2] : {
      | 	send(Situation2);
      | }
  (junction 76) -> (junction 19) : {
      | 	send(Situation1);
      | }
  (junction 27) -> (junction 33) [order 2] : {
      | 	send(Situation4);
      | }
  (junction 29) -> (junction 31) : {
      | 	send(Situation3);
      | }
  (junction 31) -> (junction 33)
  (junction 25) -> (junction 31)
  (junction 19) -> (junction 25)
  (junction 33) -> (junction 86)
  data: vx input; axAbsObj input
  events: Situation1 output FUNCTION_CALL_EVENT; Situation2 output FUNCTION_CALL_EVENT; Situation3 output FUNCTION_CALL_EVENT; Situation4 output FUNCTION_CALL_EVENT

== stateflow chart TosAeb/FcwAebJa_Step_sys/ALG_AEBSystem/ALG_AEBController/AEB_LongTgtInfoCalc/LongObjCalcInfoCalc/AEBS_LongCollisionAssesApp/LongCollisionAvoidAssess/HighLvlBrkCalc/HighLvlBrkCalc4Warning/accelAvoidHighLvlBrkSituation2/accelAvoidHighLvlBrkCalc/If Action Subsystem1/accelAvoidLowLvlBrkCalc1/AccelAvoidSituationJudge ==
  (default) -> (junction 16)
  (junction 16) -> (junction 17) [order 1] : [vxRelTargetObjCalc < 0 &&...
      |  axAbsTargetObjCalc  < 0]
  (junction 16) -> (junction 21) [order 2]
  (junction 17) -> (junction 19) : {send(Situation1);}
  (junction 21) -> (junction 23) [order 1] : [vxRelTargetObjCalc < 0 &&...
      |  axAbsTargetObjCalc  >= 0]
  (junction 21) -> (junction 27) [order 2]
  (junction 23) -> (junction 25) : {send(Situation2);}
  (junction 27) -> (junction 29) [order 1] : [vxRelTargetObjCalc > 0 &&...
      |  axAbsTargetObjCalc  < 0]
  (junction 27) -> (junction 33) [order 2] : {send(Situation4);}
  (junction 29) -> (junction 31) : {send(Situation3);}
  (junction 31) -> (junction 33)
  (junction 25) -> (junction 31)
  (junction 19) -> (junction 25)
  (junction 33) -> (junction 39)
  data: vxRelTargetObjCalc input; axAbsTargetObjCalc input
  events: Situation1 output FUNCTION_CALL_EVENT; Situation2 output FUNCTION_CALL_EVENT; Situation3 output FUNCTION_CALL_EVENT; Situation4 output FUNCTION_CALL_EVENT

== stateflow chart TosAeb/FcwAebJa_Step_sys/ALG_AEBSystem/ALG_AEBController/AEB_LongTgtInfoCalc/LongObjCalcInfoCalc/AEBS_LongCollisionAssesApp/LongCollisionAvoidAssess/HighLvlBrkCalc/HighLvlBrkCalc4Warning/accelAvoidHighLvlBrkSituation2/accelAvoidHighLvlBrkCalc/tObjStopCalc/StopTimeFlow ==
  (default) -> (junction 2)
  (junction 2) -> (junction 3) [order 1] : [accelRel == 0]
  (junction 2) -> (junction 14) [order 2]
  (junction 3) -> (junction 5)
  (junction 14) -> (junction 16) [order 1] : [(accelRel > 0 && velRel < 0)||...
      | (accelRel < 0 && velRel >= 0)]
  (junction 5) -> (junction 7) [order 1] : [velRel == 0]
  (junction 14) -> (junction 20) [order 2] : {
      | 	tStop = CNSTOPNULL;
      |  }
  (junction 16) -> (junction 18) : {
      | 	tStopFuncCalc;
      | 	tStop = tStopIn;
      | }
  (junction 5) -> (junction 11) [order 2] : {
      | 	tStop = CNSTOPMAX;
      | }
  (junction 7) -> (junction 9) : {
      | 	tStop = CNSTOPNULL;
      | }
  (junction 18) -> (junction 20)
  (junction 11) -> (junction 18)
  (junction 9) -> (junction 11)
  (junction 20) -> (junction 24)
  data: tStop output single; CNSTOPNULL constant single; CNSTOPMAX constant single; accelRel input; velRel input; tStopIn input
  events: tStopFuncCalc output FUNCTION_CALL_EVENT

== stateflow chart TosAeb/FcwAebJa_Step_sys/ALG_AEBSystem/ALG_AEBController/AEB_LongTgtInfoCalc/LongObjCalcInfoCalc/AEBS_LongCollisionAssesApp/LongCollisionAvoidAssess/HighLvlBrkCalc/HighLvlBrkCalc4Warning/accelAvoidHighLvlBrkSituation3/If Action Subsystem1/accelAvoidHighLvlBrkCalc/accelAvoidLowLvlBrkCalc/AccelAvoidSituationJudge ==
  (default) -> (junction 16)
  (junction 16) -> (junction 17) [order 1] : [vx < 0]
  (junction 16) -> (junction 27) [order 2]
  (junction 17) -> (junction 74)
  (junction 74) -> (junction 76) [order 1] : [axAbsObj  < 0]
  (junction 27) -> (junction 29) [order 1] : [ axAbsObj  < 0]
  (junction 74) -> (junction 25) [order 2] : {
      | 	send(Situation2);
      | }
  (junction 76) -> (junction 19) : {
      | 	send(Situation1);
      | }
  (junction 27) -> (junction 33) [order 2] : {
      | 	send(Situation4);
      | }
  (junction 29) -> (junction 31) : {
      | 	send(Situation3);
      | }
  (junction 31) -> (junction 33)
  (junction 25) -> (junction 31)
  (junction 19) -> (junction 25)
  (junction 33) -> (junction 86)
  data: vx input; axAbsObj input
  events: Situation1 output FUNCTION_CALL_EVENT; Situation2 output FUNCTION_CALL_EVENT; Situation3 output FUNCTION_CALL_EVENT; Situation4 output FUNCTION_CALL_EVENT

== stateflow chart TosAeb/FcwAebJa_Step_sys/ALG_AEBSystem/ALG_AEBController/AEB_LongTgtInfoCalc/LongObjCalcInfoCalc/AEBS_LongCollisionAssesApp/LongCollisionAvoidAssess/HighLvlBrkCalc/HighLvlBrkCalc4Warning/accelAvoidHighLvlBrkSituation3/If Action Subsystem1/accelAvoidHighLvlBrkCalc/accelAvoidLowLvlBrkCalc1/AccelAvoidSituationJudge ==
  (default) -> (junction 16)
  (junction 16) -> (junction 17) [order 1] : [vxRelTargetObjCalc < 0 &&...
      |  axAbsTargetObjCalc  < 0]
  (junction 16) -> (junction 21) [order 2]
  (junction 17) -> (junction 19) : {send(Situation1);}
  (junction 21) -> (junction 23) [order 1] : [vxRelTargetObjCalc < 0 &&...
      |  axAbsTargetObjCalc  >= 0]
  (junction 21) -> (junction 27) [order 2]
  (junction 23) -> (junction 25) : {send(Situation2);}
  (junction 27) -> (junction 29) [order 1] : [vxRelTargetObjCalc > 0 &&...
      |  axAbsTargetObjCalc  < 0]
  (junction 27) -> (junction 33) [order 2] : {send(Situation4);}
  (junction 29) -> (junction 31) : {send(Situation3);}
  (junction 31) -> (junction 33)
  (junction 25) -> (junction 31)
  (junction 19) -> (junction 25)
  (junction 33) -> (junction 39)
  data: vxRelTargetObjCalc input; axAbsTargetObjCalc input
  events: Situation1 output FUNCTION_CALL_EVENT; Situation2 output FUNCTION_CALL_EVENT; Situation3 output FUNCTION_CALL_EVENT; Situation4 output FUNCTION_CALL_EVENT

== stateflow chart TosAeb/FcwAebJa_Step_sys/ALG_AEBSystem/ALG_AEBController/AEB_LongTgtInfoCalc/LongObjCalcInfoCalc/AEBS_LongCollisionAssesApp/LongCollisionAvoidAssess/HighLvlBrkCalc/HighLvlBrkCalc4Warning/accelAvoidHighLvlBrkSituationJudge ==
  note: NOTE:
      | Stuation1:	tReaction == 0
      | Situation2:	tReaction < min(tEgoStop,tObjStop)
      | Situation3:	tObjStop<tReaction<=tEgoStop
      | Stuation4:	tEgoStop<tReaction<=tObjStop
      | Situation5: tReaction > max(tEgoStop,tObjStop)
  (default) -> (junction 2)
  (junction 2) -> (junction 3) [order 1] : [tReaction == 0]
  (junction 2) -> (junction 7) [order 2]
  (junction 3) -> (junction 5) : {send(Situation1);}
  (junction 7) -> (junction 9) [order 1] : [tReaction<=tEgoStop &&...
      | tReaction<=tObjStop]
  (junction 7) -> (junction 13) [order 2]
  (junction 9) -> (junction 11) : {send(Situation2);}
  (junction 13) -> (junction 15) [order 1] : [tReaction<=tEgoStop && ...
      | tReaction>tObjStop]
  (junction 13) -> (junction 19) [order 2]
  (junction 15) -> (junction 17) : {send(Situation3);}
  (junction 19) -> (junction 21) [order 1] : [tReaction>tEgoStop && ...
      | tReaction<=tObjStop ]
  (junction 19) -> (junction 25) [order 2] : {send(Situation5);}
  (junction 21) -> (junction 23) : {send(Situation4);}
  (junction 23) -> (junction 25)
  (junction 17) -> (junction 23)
  (junction 11) -> (junction 17)
  (junction 5) -> (junction 11)
  (junction 25) -> (junction 28)
  data: tEgoStop input; tObjStop input; tReaction input
  events: Situation1 output FUNCTION_CALL_EVENT; Situation2 output FUNCTION_CALL_EVENT; Situation3 output FUNCTION_CALL_EVENT; Situation4 output FUNCTION_CALL_EVENT; Situation5 output FUNCTION_CALL_EVENT

== stateflow chart TosAeb/FcwAebJa_Step_sys/ALG_AEBSystem/ALG_AEBController/AEB_LongTgtInfoCalc/LongObjCalcInfoCalc/AEBS_LongCollisionAssesApp/LongCollisionAvoidAssess/LowLvlBrkCalc/accelAvoidLowLvlBrkCalc/AccelAvoidSituationJudge ==
  (default) -> (junction 16)
  (junction 16) -> (junction 17) [order 1] : [vx < 0]
  (junction 16) -> (junction 27) [order 2]
  (junction 17) -> (junction 74)
  (junction 74) -> (junction 76) [order 1] : [axAbsObj  < 0]
  (junction 27) -> (junction 29) [order 1] : [ axAbsObj  < 0]
  (junction 74) -> (junction 25) [order 2] : {
      | 	send(Situation2);
      | }
  (junction 76) -> (junction 19) : {
      | 	send(Situation1);
      | }
  (junction 27) -> (junction 33) [order 2] : {
      | 	send(Situation4);
      | }
  (junction 29) -> (junction 31) : {
      | 	send(Situation3);
      | }
  (junction 31) -> (junction 33)
  (junction 25) -> (junction 31)
  (junction 19) -> (junction 25)
  (junction 33) -> (junction 86)
  data: vx input; axAbsObj input
  events: Situation1 output FUNCTION_CALL_EVENT; Situation2 output FUNCTION_CALL_EVENT; Situation3 output FUNCTION_CALL_EVENT; Situation4 output FUNCTION_CALL_EVENT

== stateflow chart TosAeb/FcwAebJa_Step_sys/ALG_AEBSystem/ALG_AEBController/AEB_LongTgtInfoCalc/LongObjCalcInfoCalc/AEBS_LongCollisionAssesApp/LongCollisionSeverityCalc/LongCollisionSeverityCalcFlow ==
  (default) -> (junction 2)
  (junction 2) -> (junction 3) [order 1] : [ObjectID == CNobjInvalidID]
  (junction 2) -> (junction 8) [order 2]
  (junction 3) -> (junction 5) : {tempLongCollisionSeverity = ...
      | CollisionSeverity.None;}
  (junction 8) -> (junction 10) [order 1] : [collisionSeverityHighFlg == true]
  (junction 8) -> (junction 14) [order 2]
  (junction 10) -> (junction 12) : {tempLongCollisionSeverity = ...
      | CollisionSeverity.High;}
  (junction 14) -> (junction 16) [order 1] : [collisionSeverityMediumFlg == true]
  (junction 14) -> (junction 20) [order 2]
  (junction 16) -> (junction 18) : {tempLongCollisionSeverity = ...
      | CollisionSeverity.Medium;}
  (junction 20) -> (junction 22) [order 1] : [collisionSeverityLowFlg == true]
  (junction 20) -> (junction 26) [order 2] : {tempLongCollisionSeverity = ...
      | CollisionSeverity.None;}
  (junction 22) -> (junction 24) : {tempLongCollisionSeverity = ...
      | CollisionSeverity.Low;}
  (junction 12) -> (junction 18)
  (junction 5) -> (junction 12)
  (junction 18) -> (junction 24)
  (junction 24) -> (junction 26)
  (junction 26) -> (junction 32) : {longCollisionSeverity = tempLongCollisionSeverity;}
  data: collisionSeverityLowFlg input; collisionSeverityMediumFlg input; CNobjInvalidID parameter uint8; collisionSeverityHighFlg input; ObjectID input; longCollisionSeverity output; tempLongCollisionSeverity local Enum: CollisionSeverity

== stateflow chart TosAeb/FcwAebJa_Step_sys/ALG_AEBSystem/ALG_AEBInput/LatObjInfoMap/latObjInfoMap/ObjType_Subsystem/ObjectType ==
  (default) -> (junction 17)
  (junction 17) -> (junction 18) [order 1] : [ObjCar==1]
  (junction 17) -> (junction 19) [order 2]
  (junction 18) -> (junction 23) : {ObjType = 2;}
  (junction 19) -> (junction 20) [order 1] : [ObjTruck ==1]
  (junction 19) -> (junction 26) [order 2]
  (junction 20) -> (junction 22) : {
      | 	ObjType=3;
      | }
  (junction 26) -> (junction 27) [order 1] : [ObjPed==1]
  (junction 26) -> (junction 83) [order 2]
  (junction 27) -> (junction 29) : {
      | 	ObjType=6;
      | }
  (junction 83) -> (junction 85) [order 1] : [ObjMotor==1]
  (junction 83) -> (junction 91) [order 2]
  (junction 85) -> (junction 87) : {ObjType=4;}
  (junction 91) -> (junction 93) [order 1] : [ObjBicycle==1]
  (junction 91) -> (junction 100) [order 2]
  (junction 93) -> (junction 95) : {ObjType=5;}
  (junction 100) -> (junction 102) [order 1] : [ObjGeneral==1]
  (junction 100) -> (junction 21) [order 2] : {ObjType=1;}
  (junction 102) -> (junction 104) : {ObjType=7;}
  (junction 104) -> (junction 21)
  (junction 95) -> (junction 104)
  (junction 87) -> (junction 95)
  (junction 29) -> (junction 87)
  (junction 22) -> (junction 29)
  (junction 23) -> (junction 22)
  (junction 21) -> (junction 25)
  data: ObjCar input; ObjTruck input; ObjPed input; ObjType output uint8; ObjMotor input; ObjBicycle input; ObjGeneral input; Unknown input

== stateflow chart TosAeb/FcwAebJa_Step_sys/ALG_AEBSystem/ALG_AEBController/AEB_LongTgtInfoCalc/LongObjCalcInfoCalc/AEBS_LongCollisionAssesApp/TTCCalc/TTCCalc/Situation2/SloveTTCQuadraticEquation/AccelRelEqualsZero/Chart ==
  state tempTTC = TTCCalc(distRel,velRel) [function]
  (default) -> (junction 17)
  (junction 17) -> (junction 18) [order 1] : [velRel >= 0 && distRel >= 0]
  (junction 17) -> (junction 19) [order 2]
  (junction 18) -> (junction 23) : {
      | 	TTC = CNSTOPMAX;
      | }
  (junction 19) -> (junction 20) [order 1] : [velRel <= 0 && distRel <= 0]
  (junction 19) -> (junction 21) [order 2] : {
      | 	 TTC = TTCCalc(distRel,velRel);
      | }
  (junction 20) -> (junction 22) : {
      | 	TTC = CNNull;
      | }
  (junction 22) -> (junction 21)
  (junction 23) -> (junction 22)
  (junction 21) -> (junction 24)
  data: distRel function_input; velRel function_input; tempTTC function_output; TTC output; CNSTOPMAX parameter; CNNull parameter; distRel input; velRel input
